simulink model "ClimaAdjmtOfTotFlowMgr"  (R2022b)
settings: Solver=FixedStepDiscrete  StartTime=0.0  StopTime=10.0  FixedStep=1  RelTol=1e-3
settings Model: Creator=blue.ge  LastModifiedBy=blue.ge
settings DataIOCC: SaveFormat=StructureWithTime
settings OptimizationCC: DefaultParameterBehavior=Inlined  EnhancedBackFolding=on  PassReuseOutputArgsAs=Individual arguments  ZeroExternalMemoryAtStartup=off  ZeroInternalMemoryAtStartup=off  OptimizeBlockOrder=speed  DifferentSizesBufferReuse=on  OptimizationCustomize=off  EfficientTunableParamExpr=on
settings DebuggingCC: ParameterTunabilityLossMsg=error
settings ModelReferenceCC: ModelReferenceNumInstancesAllowed=Single
settings SFSimCC: MATLABDynamicMemAlloc=off
settings RTWCC: Description=AUTOSAR  SystemTargetFile=autosar.tlc  GenCodeOnly=on  TemplateMakefile=ert_default_tmf
settings CodeAppCC: ForceParamTrailComments=on  IgnoreCustomStorageClasses=off  OperatorAnnotations=on  SimulinkDataObjDesc=on  SFDataObjDesc=on  InsertBlockDesc=on
settings STFCustomTargetCC: TargetLangStandard=C99 (ISO)  MultiwordLength=256  ParMdlRefBuildCompliant=on  CompOptLevelCompliant=on  IncludeMdlTerminateFcn=off  CombineOutputUpdateFcns=on  SuppressErrorStatus=on  ERTCustomFileBanners=on  SupportNonFinite=off  SupportComplex=off  SupportContinuousTime=off  SupportNonInlinedSFcns=off  RemoveResetFunc=on  MATLABClassNameForMDSCustomization=Simulink.SoftwareTarget.ERTCustomization  AutosarCompliant=on  UseToolchainInfoCompliant=on  LookupTableObjectStructAxisOrder=2,1,3,4,...  LUTObjectStructOrderEvenSpacing=Size,Table,Breakpoints  SystemTargetFile=autosar.tlc
settings ERTTargetCC: TargetLangStandard=C99 (ISO)  ConcurrentExecutionCompliant=off  IncludeMdlTerminateFcn=off  SuppressErrorStatus=on  SupportNonFinite=off  SupportComplex=off  ModelStepFunctionPrototypeControlCompliant=off  CPPClassGenCompliant=off  AutosarCompliant=on  LookupTableObjectStructAxisOrder=2,1,3,4,...  LUTObjectStructOrderEvenSpacing=Size,Table,Breakpoints  InlinedParameterPlacement=NonHierarchical  ERTCustomFileTemplate=example_file_process.tlc

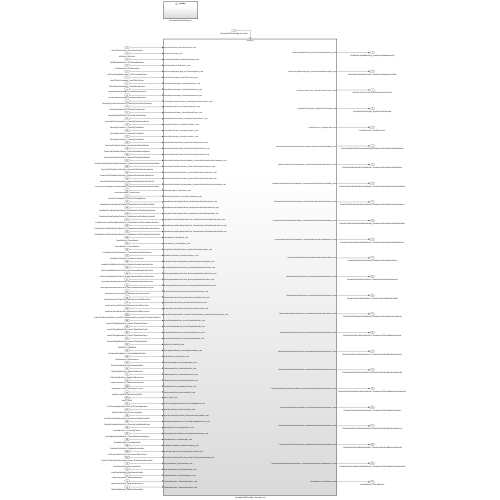
[thumbnail: Simulink's stored preview of the top-level diagram]
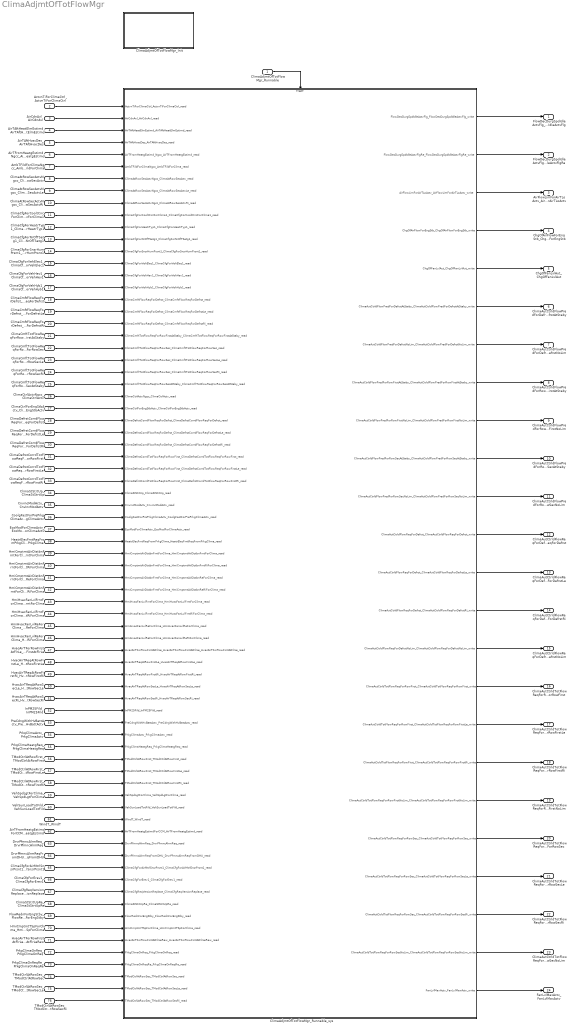
== system ClimaAdjmtOfTotFlowMgr ==
  ClimaAdjmtOfTotFlowMgr_Runnable : Inport  SampleTime=1  OutputFunctionCall=on
  ActvnTiForClimaCtrl_ActvnTiForClimaCtrl : Inport  Port=2  OutDataTypeStr=Ti33  PortDimensions=1
  AirCdnAvl_AirCdnAvl : Inport  Port=3  OutDataTypeStr=Boolean  PortDimensions=1
  AirTAftHeatElmEstimd_AirTAftHeatElmEstimd : Inport  Port=4  OutDataTypeStr=T30  PortDimensions=1
  AirTAftHvacDes_AirTAftHvacDes : Inport  Port=5  OutDataTypeStr=T30  PortDimensions=1
  AirTFromHeatgEstimd_Ngcc_AirTFromHeatgEstimd : Inport  Port=6  OutDataTypeStr=T30  PortDimensions=1
  AmbTFildForClimaNgcc_AmbTFildForClima : Inport  Port=7  OutDataTypeStr=T30  PortDimensions=1
  ClimaAtRowSecActvNgcc_ClimaAtRowSecActv : Inport  Port=8  OutDataTypeStr=Boolean  PortDimensions=1
  ClimaAtRowSecActvNgcc_ClimaAtRowSecActvLe : Inport  Port=9  OutDataTypeStr=Boolean  PortDimensions=1
  ClimaAtRowSecActvNgcc_ClimaAtRowSecActvRi : Inport  Port=10  OutDataTypeStr=Boolean  PortDimensions=1
  ClimaCfgForCooltCircForClima1_ClimaCfgForCooltCircForClima1 : Inport  Port=11  OutDataTypeStr=Boolean  PortDimensions=1
  ClimaCfgForHeatrTyp1_ClimaCfgForHeatrTyp1 : Inport  Port=12  OutDataTypeStr=Enum: ClimaCfgForHeatrTyp1  PortDimensions=1
  ClimaCfgForNrOfTSetg1_ClimaCfgForNrOfTSetg1 : Inport  Port=13  OutDataTypeStr=Enum: ClimaCfgForNrOfTSetg1  PortDimensions=1
  ClimaCfgForSnsrHumPrsnt1_ClimaCfgForSnsrHumPrsnt1 : Inport  Port=14  OutDataTypeStr=Boolean  PortDimensions=1
  ClimaCfgForVehElec1_ClimaCfgForVehElec1 : Inport  Port=15  OutDataTypeStr=Boolean  PortDimensions=1
  ClimaCfgForVehHev1_ClimaCfgForVehHev1 : Inport  Port=16  OutDataTypeStr=Boolean  PortDimensions=1
  ClimaCfgForVehHyb1_ClimaCfgForVehHyb1 : Inport  Port=17  OutDataTypeStr=Boolean  PortDimensions=1
  ClimaCmftFlowReqForDefrst_ClimaCmftFlowReqForDefrst : Inport  Port=18  OutDataTypeStr=AirFlow30  PortDimensions=1
  ClimaCmftFlowReqForDefrst_ClimaCmftFlowReqForDefrstLe : Inport  Port=19  OutDataTypeStr=AirFlow30  PortDimensions=1
  ClimaCmftFlowReqForDefrst_ClimaCmftFlowReqForDefrstRi : Inport  Port=20  OutDataTypeStr=AirFlow30  PortDimensions=1
  ClimaCmftTotFlowReqForRowFirstAtStaby_ClimaCmftTotFlowReqForRowFirstAtStaby : Inport  Port=21  OutDataTypeStr=AirFlow30  PortDimensions=1
  ClimaCmftTotFlowReqForRowSec_ClimaCmftTotFlowReqForRowSec : Inport  Port=22  OutDataTypeStr=AirFlow30  PortDimensions=1
  ClimaCmftTotFlowReqForRowSec_ClimaCmftTotFlowReqForRowSecLe : Inport  Port=23  OutDataTypeStr=AirFlow30  PortDimensions=1
  ClimaCmftTotFlowReqForRowSec_ClimaCmftTotFlowReqForRowSecRi : Inport  Port=24  OutDataTypeStr=AirFlow30  PortDimensions=1
  ClimaCmftTotFlowReqForRowSecAtStaby_ClimaCmftTotFlowReqForRowSecAtStaby : Inport  Port=25  OutDataTypeStr=AirFlow30  PortDimensions=1
  ClimaCtrlActvNgcc_ClimaCtrlActv : Inport  Port=26  OutDataTypeStr=Boolean  PortDimensions=1
  ClimaCtrlForEngStbActv_ClimaCtrlForEngStbActv : Inport  Port=27  OutDataTypeStr=Boolean  PortDimensions=1
  ClimaDefrstCorrdFlowReqForDefrst_ClimaDefrstCorrdFlowReqForDefrst : Inport  Port=28  OutDataTypeStr=AirFlow30  PortDimensions=1
  ClimaDefrstCorrdFlowReqForDefrst_ClimaDefrstCorrdFlowReqForDefrstLe : Inport  Port=29  OutDataTypeStr=AirFlow30  PortDimensions=1
  ClimaDefrstCorrdFlowReqForDefrst_ClimaDefrstCorrdFlowReqForDefrstRi : Inport  Port=30  OutDataTypeStr=AirFlow30  PortDimensions=1
  ClimaDefrstCorrdTotFlowReqForRowFirst_ClimaDefrstCorrdTotFlowReqForRowFirst : Inport  Port=31  OutDataTypeStr=AirFlow30  PortDimensions=1
  ClimaDefrstCorrdTotFlowReqForRowFirst_ClimaDefrstCorrdTotFlowReqForRowFirstLe : Inport  Port=32  OutDataTypeStr=AirFlow30  PortDimensions=1
  ClimaDefrstCorrdTotFlowReqForRowFirst_ClimaDefrstCorrdTotFlowReqForRowFirstRi : Inport  Port=33  OutDataTypeStr=AirFlow30  PortDimensions=1
  ClimaStStrtUp_ClimaStStrtUp : Inport  Port=34  OutDataTypeStr=Enum: ClimaStStrtUp  PortDimensions=1
  CnvincModActv_CnvincModActv : Inport  Port=35  OutDataTypeStr=Boolean  PortDimensions=1
  CoolgFastForPrePrkgClimaActv_CoolgFastForPrePrkgClimaActv : Inport  Port=36  OutDataTypeStr=Boolean  PortDimensions=1
  EcoModForClimaActv_EcoModForClimaActv : Inport  Port=37  OutDataTypeStr=Enum: OnOff1  PortDimensions=1
  HeatrElecFrntReqFromPrkgClima_HeatrElecFrntReqFromPrkgClima : Inport  Port=38  OutDataTypeStr=Boolean  PortDimensions=1
  HmiCmptmtAirDistbnFrntForClima_HmiCmptmtAirDistbnFrntForClima : Inport  Port=39  OutDataTypeStr=Enum: HmiCmptmtAirDistbnFrnt  PortDimensions=1
  HmiCmptmtAirDistbnFrntForClima_HmiCmptmtAirDistbnFrntRiForClima : Inport  Port=40  OutDataTypeStr=Enum: HmiCmptmtAirDistbnFrnt  PortDimensions=1
  HmiCmptmtAirDistbnFrntForClima_HmiCmptmtAirDistbnReForClima : Inport  Port=41  OutDataTypeStr=Enum: HmiCmptmtAirDistbnFrnt  PortDimensions=1
  HmiCmptmtAirDistbnFrntForClima_HmiCmptmtAirDistbnReRiForClima : Inport  Port=42  OutDataTypeStr=Enum: HmiCmptmtAirDistbnFrnt  PortDimensions=1
  HmiHvacFanLvlFrntForClima_HmiHvacFanLvlFrntForClima : Inport  Port=43  OutDataTypeStr=Enum: HmiHvacFanLvl  PortDimensions=1
  HmiHvacFanLvlFrntForClima_HmiHvacFanLvlFrntRiForClima : Inport  Port=44  OutDataTypeStr=Enum: HmiHvacFanLvl  PortDimensions=1
  HmiHvacFanLvlReForClima_HmiHvacFanLvlReForClima : Inport  Port=45  OutDataTypeStr=Enum: HmiHvacFanLvl  PortDimensions=1
  HmiHvacFanLvlReForClima_HmiHvacFanLvlReRiForClima : Inport  Port=46  OutDataTypeStr=Enum: HmiHvacFanLvl  PortDimensions=1
  HvacAirTForRowFirstAtFlrLe_HvacAirTForRowFirstAtFlrLe_HvacAirTForRowFirstAtFlrLe : Inport  Port=47  OutDataTypeStr=Bus: HvacAirTForRowFirstAtFlrLe  PortDimensions=1  BusOutputAsStruct=on
  HvacAirTReqAtRowFirstLe_HvacAirTReqAtRowFirstLe : Inport  Port=48  OutDataTypeStr=T30  PortDimensions=1
  HvacAirTReqAtRowFirstRi_HvacAirTReqAtRowFirstRi : Inport  Port=49  OutDataTypeStr=T30  PortDimensions=1
  HvacAirTReqAtRowSecLe_HvacAirTReqAtRowSecLe : Inport  Port=50  OutDataTypeStr=T30  PortDimensions=1
  HvacAirTReqAtRowSecRi_HvacAirTReqAtRowSecRi : Inport  Port=51  OutDataTypeStr=T30  PortDimensions=1
  InPM25Fild_InPM25Fild : Inport  Port=52  OutDataTypeStr=UInt10  PortDimensions=1
  PreCdngWithHvBattActv_PreCdngWithHvBattActv : Inport  Port=53  OutDataTypeStr=Boolean  PortDimensions=1
  PrkgClimaActv_PrkgClimaActv : Inport  Port=54  OutDataTypeStr=Enum: PrkgClimaActv  PortDimensions=1
  PrkgClimaHeatgReq_PrkgClimaHeatgReq : Inport  Port=55  OutDataTypeStr=Boolean  PortDimensions=1
  TModCtrlAtRowFirst_TModCtrlAtRowFirst : Inport  Port=56  OutDataTypeStr=T31  PortDimensions=1
  TModCtrlAtRowFirst_TModCtrlAtRowFirstLe : Inport  Port=57  OutDataTypeStr=T31  PortDimensions=1
  TModCtrlAtRowFirst_TModCtrlAtRowFirstRi : Inport  Port=58  OutDataTypeStr=T31  PortDimensions=1
  VehSpdLgtForClima_VehSpdLgtForClima : Inport  Port=59  OutDataTypeStr=Bus: VehSpdLgtForClima  PortDimensions=1  BusOutputAsStruct=on
  VehSunLoadTotFild_VehSunLoadTotFild : Inport  Port=60  OutDataTypeStr=SunLoad30  PortDimensions=1
  WindT_WindT : Inport  Port=61  OutDataTypeStr=Bus: WindT  PortDimensions=1  BusOutputAsStruct=on
  AirTFromHeatgEstimdForCCM_AirTFromHeatgEstimd : Inport  Port=62  OutDataTypeStr=T30  PortDimensions=1
  DrvrPfmncAlrmReq_DrvrPfmncAlrmReq : Inport  Port=63  OutDataTypeStr=Enum: DrvrPfmncWarnReq  PortDimensions=1
  DrvrPfmncAlrmReqFromDHU_DrvrPfmncAlrmReqFromDHU : Inport  Port=64  OutDataTypeStr=Enum: DrvrPfmncWarnReq  PortDimensions=1
  ClimaCfgForAirMtrlSnsrPrsnt1_ClimaCfgForAirMtrlSnsrPrsnt1 : Inport  Port=65  OutDataTypeStr=Enum: CarCfgForAirMtrlSnsrGly  PortDimensions=1
  ClimaCfgForErev1_ClimaCfgForErev1 : Inport  Port=66  OutDataTypeStr=Boolean  PortDimensions=1
  ClimaCfgReqVersionReplace_ClimaCfgReqVersionReplace : Inport  Port=67  OutDataTypeStr=UInt8  PortDimensions=1
  ClimaStStrtUpRe_ClimaStStrtUpRe : Inport  Port=68  OutDataTypeStr=Enum: ClimaStStrtUp  PortDimensions=1
  FlowRednForEngStby_FlowRednForEngStby : Inport  Port=69  OutDataTypeStr=Boolean  PortDimensions=1
  HmiCmptmtTSpForClima_HmiCmptmtTSpForClima : Inport  Port=70  OutDataTypeStr=Bus: HmiCmptmtTSp  PortDimensions=1  BusOutputAsStruct=on
  HvacAirTForRowFirstAtFlrLeRaw_HvacAirTForRowFirstAtFlrLeRaw : Inport  Port=71  OutDataTypeStr=Bus: HvacAirTForRowFirstAtFlrLe  PortDimensions=1  BusOutputAsStruct=on
  PrkgClimaOnReq_PrkgClimaOnReq : Inport  Port=72  OutDataTypeStr=Boolean  PortDimensions=1
  PrkgClimaOnReqRe_PrkgClimaOnReqRe : Inport  Port=73  OutDataTypeStr=Enum: OnOff1  PortDimensions=1
  TModCtrlAtRowSec_TModCtrlAtRowSec : Inport  Port=74  OutDataTypeStr=T31  PortDimensions=1
  TModCtrlAtRowSec_TModCtrlAtRowSecLe : Inport  Port=75  OutDataTypeStr=T31  PortDimensions=1
  TModCtrlAtRowSec_TModCtrlAtRowSecRi : Inport  Port=76  OutDataTypeStr=T31  PortDimensions=1
  ClimaAdjmtOfTotFlowMgr_Init : Subsystem
  ClimaAdjmtOfTotFlowMgr_Runnable_sys : Subsystem
  FlowDecDurgSpdIdleActvFlg_FlowDecDurgSpdIdleActvFlg : Outport  OutDataTypeStr=Boolean  PortDimensions=1
  FlowDecDurgSpdIdleActvFlgRe_FlowDecDurgSpdIdleActvFlgRe : Outport  Port=2  OutDataTypeStr=Boolean  PortDimensions=1
  AirFlowLimForAirTLoActv_AirFlowLimForAirTLoActv : Outport  Port=3  OutDataTypeStr=Boolean  PortDimensions=1
  ChgOfAirFlowForEngStb_ChgOfAirFlowForEngStb : Outport  Port=4  OutDataTypeStr=Boolean  PortDimensions=1
  ChgOfFanLvlAut_ChgOfFanLvlAut : Outport  Port=5  OutDataTypeStr=Boolean  PortDimensions=1
  ClimaAutCtrldFlowPredForDefrstAtStaby_ClimaAutCtrldFlowPredForDefrstAtStaby : Outport  Port=6  OutDataTypeStr=AirFlow30  PortDimensions=1
  ClimaAutCtrldFlowPredForDefrstNoLim_ClimaAutCtrldFlowPredForDefrstNoLim : Outport  Port=7  OutDataTypeStr=AirFlow30  PortDimensions=1
  ClimaAutCtrldFlowPredForRowFirstAtStaby_ClimaAutCtrldFlowPredForRowFirstAtStaby : Outport  Port=8  OutDataTypeStr=AirFlow30  PortDimensions=1
  ClimaAutCtrldFlowPredForRowFirstNoLim_ClimaAutCtrldFlowPredForRowFirstNoLim : Outport  Port=9  OutDataTypeStr=AirFlow30  PortDimensions=1
  ClimaAutCtrldFlowPredForRowSecAtStaby_ClimaAutCtrldFlowPredForRowSecAtStaby : Outport  Port=10  OutDataTypeStr=AirFlow30  PortDimensions=1
  ClimaAutCtrldFlowPredForRowSecNoLim_ClimaAutCtrldFlowPredForRowSecNoLim : Outport  Port=11  OutDataTypeStr=AirFlow30  PortDimensions=1
  ClimaAutCtrldFlowReqForDefrst_ClimaAutCtrldFlowReqForDefrst : Outport  Port=12  OutDataTypeStr=AirFlow30  PortDimensions=1
  ClimaAutCtrldFlowReqForDefrst_ClimaAutCtrldFlowReqForDefrstLe : Outport  Port=13  OutDataTypeStr=AirFlow30  PortDimensions=1
  ClimaAutCtrldFlowReqForDefrst_ClimaAutCtrldFlowReqForDefrstRi : Outport  Port=14  OutDataTypeStr=AirFlow30  PortDimensions=1
  ClimaAutCtrldFlowReqForDefrstNoLim_ClimaAutCtrldFlowReqForDefrstNoLim : Outport  Port=15  OutDataTypeStr=AirFlow30  PortDimensions=1
  ClimaAutCtrldTotFlowReqForRowFirst_ClimaAutCtrldTotFlowReqForRowFirst : Outport  Port=16  OutDataTypeStr=AirFlow30  PortDimensions=1
  ClimaAutCtrldTotFlowReqForRowFirst_ClimaAutCtrldTotFlowReqForRowFirstLe : Outport  Port=17  OutDataTypeStr=AirFlow30  PortDimensions=1
  ClimaAutCtrldTotFlowReqForRowFirst_ClimaAutCtrldTotFlowReqForRowFirstRi : Outport  Port=18  OutDataTypeStr=AirFlow30  PortDimensions=1
  ClimaAutCtrldTotFlowReqForRowFirstNoLim_ClimaAutCtrldTotFlowReqForRowFirstNoLim : Outport  Port=19  OutDataTypeStr=AirFlow30  PortDimensions=1
  ClimaAutCtrldTotFlowReqForRowSec_ClimaAutCtrldTotFlowReqForRowSec : Outport  Port=20  OutDataTypeStr=AirFlow30  PortDimensions=1
  ClimaAutCtrldTotFlowReqForRowSec_ClimaAutCtrldTotFlowReqForRowSecLe : Outport  Port=21  OutDataTypeStr=AirFlow30  PortDimensions=1
  ClimaAutCtrldTotFlowReqForRowSec_ClimaAutCtrldTotFlowReqForRowSecRi : Outport  Port=22  OutDataTypeStr=AirFlow30  PortDimensions=1
  ClimaAutCtrldTotFlowReqForRowSecNoLim_ClimaAutCtrldTotFlowReqForRowSecNoLim : Outport  Port=23  OutDataTypeStr=AirFlow30  PortDimensions=1
  FanLvlMaxActv_FanLvlMaxActv : Outport  Port=24  OutDataTypeStr=Boolean  PortDimensions=1
  wires:
  ActvnTiForClimaCtrl_ActvnTiForClimaCtrl/1 -> ClimaAdjmtOfTotFlowMgr_Runnable_sys/1(ActvnTiForClimaCtrl_ActvnTiForClimaCtrl_read)
  AirCdnAvl_AirCdnAvl/1 -> ClimaAdjmtOfTotFlowMgr_Runnable_sys/2(AirCdnAvl_AirCdnAvl_read)
  AirTAftHeatElmEstimd_AirTAftHeatElmEstimd/1 -> ClimaAdjmtOfTotFlowMgr_Runnable_sys/3(AirTAftHeatElmEstimd_AirTAftHeatElmEstimd_read)
  AirTAftHvacDes_AirTAftHvacDes/1 -> ClimaAdjmtOfTotFlowMgr_Runnable_sys/4(AirTAftHvacDes_AirTAftHvacDes_read)
  AirTFromHeatgEstimd_Ngcc_AirTFromHeatgEstimd/1 -> ClimaAdjmtOfTotFlowMgr_Runnable_sys/5(AirTFromHeatgEstimd_Ngcc_AirTFromHeatgEstimd_read)
  AmbTFildForClimaNgcc_AmbTFildForClima/1 -> ClimaAdjmtOfTotFlowMgr_Runnable_sys/6(AmbTFildForClimaNgcc_AmbTFildForClima_read)
  ClimaAtRowSecActvNgcc_ClimaAtRowSecActv/1 -> ClimaAdjmtOfTotFlowMgr_Runnable_sys/7(ClimaAtRowSecActvNgcc_ClimaAtRowSecActv_read)
  ClimaAtRowSecActvNgcc_ClimaAtRowSecActvLe/1 -> ClimaAdjmtOfTotFlowMgr_Runnable_sys/8(ClimaAtRowSecActvNgcc_ClimaAtRowSecActvLe_read)
  ClimaAtRowSecActvNgcc_ClimaAtRowSecActvRi/1 -> ClimaAdjmtOfTotFlowMgr_Runnable_sys/9(ClimaAtRowSecActvNgcc_ClimaAtRowSecActvRi_read)
  ClimaCfgForCooltCircForClima1_ClimaCfgForCooltCircForClima1/1 -> ClimaAdjmtOfTotFlowMgr_Runnable_sys/10(ClimaCfgForCooltCircForClima1_ClimaCfgForCooltCircForClima1_read)
  ClimaCfgForHeatrTyp1_ClimaCfgForHeatrTyp1/1 -> ClimaAdjmtOfTotFlowMgr_Runnable_sys/11(ClimaCfgForHeatrTyp1_ClimaCfgForHeatrTyp1_read)
  ClimaCfgForNrOfTSetg1_ClimaCfgForNrOfTSetg1/1 -> ClimaAdjmtOfTotFlowMgr_Runnable_sys/12(ClimaCfgForNrOfTSetg1_ClimaCfgForNrOfTSetg1_read)
  ClimaCfgForSnsrHumPrsnt1_ClimaCfgForSnsrHumPrsnt1/1 -> ClimaAdjmtOfTotFlowMgr_Runnable_sys/13(ClimaCfgForSnsrHumPrsnt1_ClimaCfgForSnsrHumPrsnt1_read)
  ClimaCfgForVehElec1_ClimaCfgForVehElec1/1 -> ClimaAdjmtOfTotFlowMgr_Runnable_sys/14(ClimaCfgForVehElec1_ClimaCfgForVehElec1_read)
  ClimaCfgForVehHev1_ClimaCfgForVehHev1/1 -> ClimaAdjmtOfTotFlowMgr_Runnable_sys/15(ClimaCfgForVehHev1_ClimaCfgForVehHev1_read)
  ClimaCfgForVehHyb1_ClimaCfgForVehHyb1/1 -> ClimaAdjmtOfTotFlowMgr_Runnable_sys/16(ClimaCfgForVehHyb1_ClimaCfgForVehHyb1_read)
  ClimaCmftFlowReqForDefrst_ClimaCmftFlowReqForDefrst/1 -> ClimaAdjmtOfTotFlowMgr_Runnable_sys/17(ClimaCmftFlowReqForDefrst_ClimaCmftFlowReqForDefrst_read)
  ClimaCmftFlowReqForDefrst_ClimaCmftFlowReqForDefrstLe/1 -> ClimaAdjmtOfTotFlowMgr_Runnable_sys/18(ClimaCmftFlowReqForDefrst_ClimaCmftFlowReqForDefrstLe_read)
  ClimaCmftFlowReqForDefrst_ClimaCmftFlowReqForDefrstRi/1 -> ClimaAdjmtOfTotFlowMgr_Runnable_sys/19(ClimaCmftFlowReqForDefrst_ClimaCmftFlowReqForDefrstRi_read)
  ClimaCmftTotFlowReqForRowFirstAtStaby_ClimaCmftTotFlowReqForRowFirstAtStaby/1 -> ClimaAdjmtOfTotFlowMgr_Runnable_sys/20(ClimaCmftTotFlowReqForRowFirstAtStaby_ClimaCmftTotFlowReqForRowFirstAtStaby_read)
  ClimaCmftTotFlowReqForRowSec_ClimaCmftTotFlowReqForRowSec/1 -> ClimaAdjmtOfTotFlowMgr_Runnable_sys/21(ClimaCmftTotFlowReqForRowSec_ClimaCmftTotFlowReqForRowSec_read)
  ClimaCmftTotFlowReqForRowSec_ClimaCmftTotFlowReqForRowSecLe/1 -> ClimaAdjmtOfTotFlowMgr_Runnable_sys/22(ClimaCmftTotFlowReqForRowSec_ClimaCmftTotFlowReqForRowSecLe_read)
  ClimaCmftTotFlowReqForRowSec_ClimaCmftTotFlowReqForRowSecRi/1 -> ClimaAdjmtOfTotFlowMgr_Runnable_sys/23(ClimaCmftTotFlowReqForRowSec_ClimaCmftTotFlowReqForRowSecRi_read)
  ClimaCmftTotFlowReqForRowSecAtStaby_ClimaCmftTotFlowReqForRowSecAtStaby/1 -> ClimaAdjmtOfTotFlowMgr_Runnable_sys/24(ClimaCmftTotFlowReqForRowSecAtStaby_ClimaCmftTotFlowReqForRowSecAtStaby_read)
  ClimaCtrlActvNgcc_ClimaCtrlActv/1 -> ClimaAdjmtOfTotFlowMgr_Runnable_sys/25(ClimaCtrlActvNgcc_ClimaCtrlActv_read)
  ClimaCtrlForEngStbActv_ClimaCtrlForEngStbActv/1 -> ClimaAdjmtOfTotFlowMgr_Runnable_sys/26(ClimaCtrlForEngStbActv_ClimaCtrlForEngStbActv_read)
  ClimaDefrstCorrdFlowReqForDefrst_ClimaDefrstCorrdFlowReqForDefrst/1 -> ClimaAdjmtOfTotFlowMgr_Runnable_sys/27(ClimaDefrstCorrdFlowReqForDefrst_ClimaDefrstCorrdFlowReqForDefrst_read)
  ClimaDefrstCorrdFlowReqForDefrst_ClimaDefrstCorrdFlowReqForDefrstLe/1 -> ClimaAdjmtOfTotFlowMgr_Runnable_sys/28(ClimaDefrstCorrdFlowReqForDefrst_ClimaDefrstCorrdFlowReqForDefrstLe_read)
  ClimaDefrstCorrdFlowReqForDefrst_ClimaDefrstCorrdFlowReqForDefrstRi/1 -> ClimaAdjmtOfTotFlowMgr_Runnable_sys/29(ClimaDefrstCorrdFlowReqForDefrst_ClimaDefrstCorrdFlowReqForDefrstRi_read)
  ClimaDefrstCorrdTotFlowReqForRowFirst_ClimaDefrstCorrdTotFlowReqForRowFirst/1 -> ClimaAdjmtOfTotFlowMgr_Runnable_sys/30(ClimaDefrstCorrdTotFlowReqForRowFirst_ClimaDefrstCorrdTotFlowReqForRowFirst_read)
  ClimaDefrstCorrdTotFlowReqForRowFirst_ClimaDefrstCorrdTotFlowReqForRowFirstLe/1 -> ClimaAdjmtOfTotFlowMgr_Runnable_sys/31(ClimaDefrstCorrdTotFlowReqForRowFirst_ClimaDefrstCorrdTotFlowReqForRowFirstLe_read)
  ClimaDefrstCorrdTotFlowReqForRowFirst_ClimaDefrstCorrdTotFlowReqForRowFirstRi/1 -> ClimaAdjmtOfTotFlowMgr_Runnable_sys/32(ClimaDefrstCorrdTotFlowReqForRowFirst_ClimaDefrstCorrdTotFlowReqForRowFirstRi_read)
  ClimaStStrtUp_ClimaStStrtUp/1 -> ClimaAdjmtOfTotFlowMgr_Runnable_sys/33(ClimaStStrtUp_ClimaStStrtUp_read)
  CnvincModActv_CnvincModActv/1 -> ClimaAdjmtOfTotFlowMgr_Runnable_sys/34(CnvincModActv_CnvincModActv_read)
  CoolgFastForPrePrkgClimaActv_CoolgFastForPrePrkgClimaActv/1 -> ClimaAdjmtOfTotFlowMgr_Runnable_sys/35(CoolgFastForPrePrkgClimaActv_CoolgFastForPrePrkgClimaActv_read)
  EcoModForClimaActv_EcoModForClimaActv/1 -> ClimaAdjmtOfTotFlowMgr_Runnable_sys/36(EcoModForClimaActv_EcoModForClimaActv_read)
  HeatrElecFrntReqFromPrkgClima_HeatrElecFrntReqFromPrkgClima/1 -> ClimaAdjmtOfTotFlowMgr_Runnable_sys/37(HeatrElecFrntReqFromPrkgClima_HeatrElecFrntReqFromPrkgClima_read)
  HmiCmptmtAirDistbnFrntForClima_HmiCmptmtAirDistbnFrntForClima/1 -> ClimaAdjmtOfTotFlowMgr_Runnable_sys/38(HmiCmptmtAirDistbnFrntForClima_HmiCmptmtAirDistbnFrntForClima_read)
  HmiCmptmtAirDistbnFrntForClima_HmiCmptmtAirDistbnFrntRiForClima/1 -> ClimaAdjmtOfTotFlowMgr_Runnable_sys/39(HmiCmptmtAirDistbnFrntForClima_HmiCmptmtAirDistbnFrntRiForClima_read)
  HmiCmptmtAirDistbnFrntForClima_HmiCmptmtAirDistbnReForClima/1 -> ClimaAdjmtOfTotFlowMgr_Runnable_sys/40(HmiCmptmtAirDistbnFrntForClima_HmiCmptmtAirDistbnReForClima_read)
  HmiCmptmtAirDistbnFrntForClima_HmiCmptmtAirDistbnReRiForClima/1 -> ClimaAdjmtOfTotFlowMgr_Runnable_sys/41(HmiCmptmtAirDistbnFrntForClima_HmiCmptmtAirDistbnReRiForClima_read)
  HmiHvacFanLvlFrntForClima_HmiHvacFanLvlFrntForClima/1 -> ClimaAdjmtOfTotFlowMgr_Runnable_sys/42(HmiHvacFanLvlFrntForClima_HmiHvacFanLvlFrntForClima_read)
  HmiHvacFanLvlFrntForClima_HmiHvacFanLvlFrntRiForClima/1 -> ClimaAdjmtOfTotFlowMgr_Runnable_sys/43(HmiHvacFanLvlFrntForClima_HmiHvacFanLvlFrntRiForClima_read)
  HmiHvacFanLvlReForClima_HmiHvacFanLvlReForClima/1 -> ClimaAdjmtOfTotFlowMgr_Runnable_sys/44(HmiHvacFanLvlReForClima_HmiHvacFanLvlReForClima_read)
  HmiHvacFanLvlReForClima_HmiHvacFanLvlReRiForClima/1 -> ClimaAdjmtOfTotFlowMgr_Runnable_sys/45(HmiHvacFanLvlReForClima_HmiHvacFanLvlReRiForClima_read)
  HvacAirTForRowFirstAtFlrLe_HvacAirTForRowFirstAtFlrLe_HvacAirTForRowFirstAtFlrLe/1 -> ClimaAdjmtOfTotFlowMgr_Runnable_sys/46(HvacAirTForRowFirstAtFlrLe_HvacAirTForRowFirstAtFlrLe_HvacAirTForRowFirstAtFlrLe_read)
  HvacAirTReqAtRowFirstLe_HvacAirTReqAtRowFirstLe/1 -> ClimaAdjmtOfTotFlowMgr_Runnable_sys/47(HvacAirTReqAtRowFirstLe_HvacAirTReqAtRowFirstLe_read)
  HvacAirTReqAtRowFirstRi_HvacAirTReqAtRowFirstRi/1 -> ClimaAdjmtOfTotFlowMgr_Runnable_sys/48(HvacAirTReqAtRowFirstRi_HvacAirTReqAtRowFirstRi_read)
  HvacAirTReqAtRowSecLe_HvacAirTReqAtRowSecLe/1 -> ClimaAdjmtOfTotFlowMgr_Runnable_sys/49(HvacAirTReqAtRowSecLe_HvacAirTReqAtRowSecLe_read)
  HvacAirTReqAtRowSecRi_HvacAirTReqAtRowSecRi/1 -> ClimaAdjmtOfTotFlowMgr_Runnable_sys/50(HvacAirTReqAtRowSecRi_HvacAirTReqAtRowSecRi_read)
  InPM25Fild_InPM25Fild/1 -> ClimaAdjmtOfTotFlowMgr_Runnable_sys/51(InPM25Fild_InPM25Fild_read)
  PreCdngWithHvBattActv_PreCdngWithHvBattActv/1 -> ClimaAdjmtOfTotFlowMgr_Runnable_sys/52(PreCdngWithHvBattActv_PreCdngWithHvBattActv_read)
  PrkgClimaActv_PrkgClimaActv/1 -> ClimaAdjmtOfTotFlowMgr_Runnable_sys/53(PrkgClimaActv_PrkgClimaActv_read)
  PrkgClimaHeatgReq_PrkgClimaHeatgReq/1 -> ClimaAdjmtOfTotFlowMgr_Runnable_sys/54(PrkgClimaHeatgReq_PrkgClimaHeatgReq_read)
  TModCtrlAtRowFirst_TModCtrlAtRowFirst/1 -> ClimaAdjmtOfTotFlowMgr_Runnable_sys/55(TModCtrlAtRowFirst_TModCtrlAtRowFirst_read)
  TModCtrlAtRowFirst_TModCtrlAtRowFirstLe/1 -> ClimaAdjmtOfTotFlowMgr_Runnable_sys/56(TModCtrlAtRowFirst_TModCtrlAtRowFirstLe_read)
  TModCtrlAtRowFirst_TModCtrlAtRowFirstRi/1 -> ClimaAdjmtOfTotFlowMgr_Runnable_sys/57(TModCtrlAtRowFirst_TModCtrlAtRowFirstRi_read)
  VehSpdLgtForClima_VehSpdLgtForClima/1 -> ClimaAdjmtOfTotFlowMgr_Runnable_sys/58(VehSpdLgtForClima_VehSpdLgtForClima_read)
  VehSunLoadTotFild_VehSunLoadTotFild/1 -> ClimaAdjmtOfTotFlowMgr_Runnable_sys/59(VehSunLoadTotFild_VehSunLoadTotFild_read)
  WindT_WindT/1 -> ClimaAdjmtOfTotFlowMgr_Runnable_sys/60(WindT_WindT_read)